simulink model "TosAeb"  (R2021a)
settings: Solver=FixedStepDiscrete  StartTime=0.0  StopTime=inf  FixedStep=0.01  RelTol=1e-3
undefined symbols (not defined in the file or in its folder): ACCModSts, AccSts1, AEB_ObjectMovingOrientation, AEB_ObjectMovingState, CN_BTCActive, CN_CurrentGearR, CN_CurrentGearR_DHT, CN_PTCActive, CN_VDCActive, CN_VSC_EspOff, CN_VSC_FCW_AEB_Error, CN_VSC_FCW_AEB_NoDispay, CN_VSC_FCW_AEB_PerfmDgdtn, CN_VSC_FCW_AEB_TempUnAvlbl, CN_VSC_VehStandstill, CNaccelAvoidMax, CNaccelAvoidMin, CNbrakePedalPress, CNcomfortStrLeftAccelMax, CNcomfortStrLeftAccelMin, CNcomfortStrRightAccelMax, CNcomfortStrRightAccelMin, CNDrvDoorOpen, CNDrvModeEco, CNDrvModeNorm, CNDrvModeOther, CNDrvModeSport, CNDrvSBRreminder, CNNull, CNobjInvalidID, CNPassDoorOpen, CNPassSBRreminder, CNPEBSAccelNegInf, CNPEBSAccelNull, CNPEBSTimeInf, CNPEBSTimeNull, CNSTOPMAX, CNSTOPNULL, CNsubjWidth, CNSwtOFF, CNSwtON, CNTTCMAX, CNTTCNULL, CNVehConfigAEBCycNone, CNVehConfigAEBNone, CNVehConfigAEBPedNone, CNVehConfigFCWNone, CNVehConfigJANone, CollisionSeverity, FALSE, FCWSnvty, JACycObjCalcInfoDefaultParams, JAPedObjCalcInfoDefaultParams, JAVehObjCalcInfoDefaultParams, latCycObjCalcInfoDefaultParams, latPedObjCalcInfoDefaultParams, longObjCalcInfoDefaultParams, ModSts, movingStatus, ObjectType ... (+1112)
libraries: simulink x3031, AEBSFunctionLib x50, Ta_Library x33, AEBSStateMachineLib x23, Mylibrary x12, simulink_extras x7, ObjSelect_lib x3, dspstat3 x2
(part 90 of 102)

== system TosAeb/AEB_Objsel/TA_Processor/AEB_ObjSelect/TargetInfoCheck/Data_precess/TA_EffectCalculate/Obj_FilterFlgCalculate/Long_ObjFilter/TTR_Calculate/CalculateTTRAndTTM_else/LongitudinalTTRComputer/CalculateTTI/StationaryObject/CheckIfSolutionExists1/SizeChecker ==
  Signal : Inport  OutDataTypeStr=DataTypeIn
      settings: AttributesFormatString=DT=%<OutDataTypeStr>
  Abs : Abs  SaturateOnIntegerOverflow=on
  LimitSource : Constant  Value=Limit  OutDataTypeStr=Inherit: Inherit via back propagation
      settings: AttributesFormatString=DT=%<OutDataTypeStr>
  Relational Operator : RelationalOperator  Operator=>  OutDataTypeStr=boolean  RndMeth=Nearest  InputSameDT=on
      settings: AttributesFormatString=InputSameDT=%<InputSameDT>
  TestOk : Outport  OutDataTypeStr=boolean
      settings: AttributesFormatString=DT=%<OutDataTypeStr>
  wires:
  Relational Operator/1 -> TestOk/1  "TestOk"
  Signal/1 -> Abs/1  "Signal"
  LimitSource/1 -> Relational Operator/2  "LimitSource"
  Abs/1 -> Relational Operator/1  "AbsoluteSignal"

== system TosAeb/AEB_Objsel/TA_Processor/AEB_ObjSelect/TargetInfoCheck/Data_precess/TA_EffectCalculate/Obj_FilterFlgCalculate/Long_ObjFilter/TTR_Calculate/CalculateTTRAndTTM_else/LongitudinalTTRComputer/CalculateTTI/StationaryObject/TimeToIntersectionComputer/Compare To Zero ==
  u : Inport
  Compare : RelationalOperator  Operator=<  OutDataTypeStr=boolean  RndMeth=Nearest  InputSameDT=on
  Constant : Constant  DisableCoverage=on  Value=0  OutDataTypeStr=Inherit: Inherit via back propagation  PreserveConstantTs=on
  y : Outport
  wires:
  Constant/1 -> Compare/2
  u/1 -> Compare/1
  Compare/1 -> y/1

== system TosAeb/AEB_Objsel/TA_Processor/AEB_ObjSelect/TargetInfoCheck/Data_precess/TA_EffectCalculate/Obj_FilterFlgCalculate/Long_ObjFilter/TTR_Calculate/CalculateTTRAndTTM_else/LongitudinalTTRComputer/CalculateTTI/StationaryObject/TimeToIntersectionComputer/SolutionChooser ==
  note: If ARel is negative, choose the largest solution.
      | Otherwise, choose the smallest solution.
  Solutions : Inport
  ARel : Inport  Port=2  OutDataTypeStr=single
      settings: AttributesFormatString=DT=%<OutDataTypeStr>
  Compare To Zero1 : Subsystem  mask(Compare To Zero){relop=<, OutDataTypeStr=boolean, ZeroCross=on}
      mask prompts: relop "Simulink:masks:Operator_MP"; OutDataTypeStr "Simulink:masks:OutputDataType_MP"; ZeroCross "Simulink:masks:EnableZerocrossingDetection_MP"
      mask help: Simulink:masks:CompareToZero_MD
      mask init: same as TosAeb/AEB_Objsel/TA_Processor/AEB_ObjSelect/TargetInfoCheck/Data_precess/TA_EffectCalculate/Obj_FilterFlgCalculate/Long_ObjFilter/TTR_Calculate/CalculateTTRAndTTM_else/ModifyTTRAtSameSpeedAndAcceleration/Compare To Zero
      mask TypeOptions relop: == | ~= | < | <= | >= | >
      mask TypeOptions OutDataTypeStr: Simulink:masks:uint8 | Simulink:masks:boolean
      mask Callback ZeroCross: sl('zeroCrossEnable', gcbh);
      mask Display: disp([relop,' 0'])
  Selector : Selector  InputPortWidth=2  IndexOptions=Index vector (dialog)  Indices=1  OutputSizes=1
  Selector1 : Selector  InputPortWidth=2  IndexOptions=Index vector (dialog)  Indices=2  OutputSizes=1
  Switch3 : Switch  Criteria=u2 ~= 0  InputSameDT=on
      settings: AttributesFormatString=InputSameDT=%<InputSameDT>
  Solution : Outport
  wires:
  Selector1/1 -> Switch3/3
  Solutions/1 -> [Solutions], Selector/1
  Selector/1 -> Switch3/1
  Switch3/1 -> Solution/1  "Solution"
  ARel/1 -> Compare To Zero1/1(u)
  Compare To Zero1/1(y) -> Switch3/2  "NegativeARel"
  [Solutions] -> Selector1/1

== system TosAeb/AEB_Objsel/TA_Processor/AEB_ObjSelect/TargetInfoCheck/Data_precess/TA_EffectCalculate/Obj_FilterFlgCalculate/Long_ObjFilter/TTR_Calculate/CalculateTTRAndTTM_else/LongitudinalTTRComputer/CalculateTTI/StationaryObject/TimeToIntersectionComputer1/Compare To Zero ==
  u : Inport
  Compare : RelationalOperator  Operator=<  OutDataTypeStr=boolean  RndMeth=Nearest  InputSameDT=on
  Constant : Constant  DisableCoverage=on  Value=0  OutDataTypeStr=Inherit: Inherit via back propagation  PreserveConstantTs=on
  y : Outport
  wires:
  Compare/1 -> y/1
  u/1 -> Compare/1
  Constant/1 -> Compare/2

== system TosAeb/AEB_Objsel/TA_Processor/AEB_ObjSelect/TargetInfoCheck/Data_precess/TA_EffectCalculate/Obj_FilterFlgCalculate/Long_ObjFilter/TTR_Calculate/CalculateTTRAndTTM_else/LongitudinalTTRComputer/CalculateTTI/StationaryObject/TimeToIntersectionComputer1/SolutionChooser ==
  note: If ARel is negative, choose the largest solution.
      | Otherwise, choose the smallest solution.
  Solutions : Inport
  ARel : Inport  Port=2  OutDataTypeStr=single
      settings: AttributesFormatString=DT=%<OutDataTypeStr>
  Compare To Zero1 : Subsystem  mask(Compare To Zero){relop=<, OutDataTypeStr=boolean, ZeroCross=on}
      mask prompts: relop "Simulink:masks:Operator_MP"; OutDataTypeStr "Simulink:masks:OutputDataType_MP"; ZeroCross "Simulink:masks:EnableZerocrossingDetection_MP"
      mask help: Simulink:masks:CompareToZero_MD
      mask init: same as TosAeb/AEB_Objsel/TA_Processor/AEB_ObjSelect/TargetInfoCheck/Data_precess/TA_EffectCalculate/Obj_FilterFlgCalculate/Long_ObjFilter/TTR_Calculate/CalculateTTRAndTTM_else/ModifyTTRAtSameSpeedAndAcceleration/Compare To Zero
      mask TypeOptions relop: == | ~= | < | <= | >= | >
      mask TypeOptions OutDataTypeStr: Simulink:masks:uint8 | Simulink:masks:boolean
      mask Callback ZeroCross: sl('zeroCrossEnable', gcbh);
      mask Display: disp([relop,' 0'])
  Selector : Selector  InputPortWidth=2  IndexOptions=Index vector (dialog)  Indices=1  OutputSizes=1
  Selector1 : Selector  InputPortWidth=2  IndexOptions=Index vector (dialog)  Indices=2  OutputSizes=1
  Switch3 : Switch  Criteria=u2 ~= 0  InputSameDT=on
      settings: AttributesFormatString=InputSameDT=%<InputSameDT>
  Solution : Outport
  wires:
  [Solutions] -> Selector1/1
  Compare To Zero1/1(y) -> Switch3/2  "NegativeARel"
  ARel/1 -> Compare To Zero1/1(u)
  Switch3/1 -> Solution/1  "Solution"
  Selector/1 -> Switch3/1
  Solutions/1 -> Selector/1, [Solutions]
  Selector1/1 -> Switch3/3

== system TosAeb/AEB_Objsel/TA_Processor/AEB_ObjSelect/TargetInfoCheck/Data_precess/TA_EffectCalculate/ValideateObj/ObjPos_Calculate/ObjPos_Calculateelse/InPath_Cal/DetermineInPath/PredictHostLateralPositionAtTTR/CalculateHeading/ExtractVelocitiesAtStartOfSegments/CheckSpeed ==
  x : Inport
  y : Inport  Port=2
  LatSpdThresholdNonVeh1 : Constant  Value=P_TA_SpdThdInAsyEvlrCritEve
  Product : Product
  Product1 : Product
  Product2 : Product
  Relational Operator : RelationalOperator  Operator=>  OutDataTypeStr=boolean  RndMeth=Nearest
  Subtract : Sum
  SpeedValid : Outport
  wires:
  y/1 -> Product/1, Product/2
  Subtract/1 -> Relational Operator/1  "VelocitySquared"
  Product1/1 -> Subtract/1
  Product/1 -> Subtract/2
  x/1 -> Product1/2, Product1/1
  LatSpdThresholdNonVeh1/1 -> Product2/1, Product2/2
  Product2/1 -> Relational Operator/2
  Relational Operator/1 -> SpeedValid/1  "SpeedValid"

== system TosAeb/AEB_Objsel/TA_Processor/AEB_ObjSelect/TargetInfoCheck/Data_precess/TA_EffectCalculate/ValideateObj/ObjPos_Calculate/ObjPos_Calculateelse/InPath_Cal/DetermineInPath/PredictHostLateralPositionAtTTR/CalculateHeading/ExtractVelocitiesAtStartOfSegments/ExtractVelocityVectorFirstPoint ==
  PathEstimn : Inport
  FirstPointInSegment : Constant  Value=single(0)
  PositionAndDerivative : MATLAB Function  TreatAsAtomicUnit=on
  PositionAndDerivative1 : MATLAB Function  TreatAsAtomicUnit=on
  SegmentComponents : Subsystem
  Terminator1 : Terminator
  Terminator2 : Terminator
  dx : Outport
  dy : Outport  Port=2
  wires:
  SegmentComponents/6(P1y) -> PositionAndDerivative1/2(P1)
  SegmentComponents/2(P1x) -> PositionAndDerivative/2(P1)
  SegmentComponents/7(P2y) -> PositionAndDerivative1/3(P2)
  SegmentComponents/1(P0x) -> PositionAndDerivative/1(P0)
  SegmentComponents/8(P3y) -> PositionAndDerivative1/4(P3)
  SegmentComponents/4(P3x) -> PositionAndDerivative/4(P3)
  SegmentComponents/3(P2x) -> PositionAndDerivative/3(P2)
  SegmentComponents/5(P0y) -> PositionAndDerivative1/1(P0)
  PathEstimn/1 -> SegmentComponents/1(Segment)
  PositionAndDerivative1/2(dy) -> dy/1
  PositionAndDerivative/1(x) -> Terminator1/1
  FirstPointInSegment/1 -> PositionAndDerivative1/5(tau), PositionAndDerivative/5(tau)
  PositionAndDerivative/2(dx) -> dx/1
  PositionAndDerivative1/1(y) -> Terminator2/1

== system TosAeb/FcwAebJa_Step_sys/ALG_AEBSystem/ALG_AEBController/AEB_LongTgtInfoCalc/LongObjCalcInfoCalc/AEBS_LongCollisionAssesApp/LongCollisionAvoidAssess/HighLvlBrkCalc/HighLvlBrkCalc4Brake/accelAvoidHighLvlBrkSituation2/accelAvoidHighLvlBrkCalc/If Action Subsystem1/accelAvoidLowLvlBrkCalc/Situation1/Case1 ==
  axAbsObj : Inport
  vx : Inport  Port=2
  dxEgoBrake : Inport  Port=3
  Action Port : ActionPort  ActionPortLabel=if(u1)
  Divide : Product  Inputs=*/
  Gain : Gain  Gain=2
  Math Function : Math  Operator=square  SignedPower=on  SaturateOnIntegerOverflow=on
  Subtract : Sum  Inputs=+-
  accelAvoid : Outport
  wires:
  vx/1 -> Math Function/1
  Math Function/1 -> Divide/1
  Gain/1 -> Divide/2
  dxEgoBrake/1 -> Gain/1
  Divide/1 -> Subtract/2
  axAbsObj/1 -> Subtract/1
  Subtract/1 -> accelAvoid/1

== system TosAeb/FcwAebJa_Step_sys/ALG_AEBSystem/ALG_AEBController/AEB_LongTgtInfoCalc/LongObjCalcInfoCalc/AEBS_LongCollisionAssesApp/LongCollisionAvoidAssess/HighLvlBrkCalc/HighLvlBrkCalc4Brake/accelAvoidHighLvlBrkSituation2/accelAvoidHighLvlBrkCalc/If Action Subsystem1/accelAvoidLowLvlBrkCalc/Situation1/Case2 ==
  note: vEgo^2
      | aAvoid = ----------------------------------
      |                   vObj^2
      |                  ---------  - 2(dRel - dScure)
      |                   aObj
  subjVel : Inport
  vxAbsObj : Inport  Port=2
  axAbsObj : Inport  Port=3
  dxEgoBrake : Inport  Port=4
  Action Port : ActionPort  ActionPortLabel=else
  Divide : Product  Inputs=*/
  Divide1 : Product  Inputs=*/
  Gain : Gain  Gain=2
  Math Function : Math  Operator=square  SignedPower=on  SaturateOnIntegerOverflow=on
  Math Function1 : Math  Operator=square  SignedPower=on  SaturateOnIntegerOverflow=on
  Subtract : Sum  Inputs=+-
  accelAvoid : Outport
  wires:
  vxAbsObj/1 -> Math Function/1
  Math Function/1 -> Divide/1
  axAbsObj/1 -> Divide/2
  Divide/1 -> Subtract/1
  dxEgoBrake/1 -> Gain/1
  Gain/1 -> Subtract/2
  subjVel/1 -> Math Function1/1
  Subtract/1 -> Divide1/2
  Math Function1/1 -> Divide1/1
  Divide1/1 -> accelAvoid/1

== system TosAeb/FcwAebJa_Step_sys/ALG_AEBSystem/ALG_AEBController/AEB_LongTgtInfoCalc/LongObjCalcInfoCalc/AEBS_LongCollisionAssesApp/LongCollisionAvoidAssess/HighLvlBrkCalc/HighLvlBrkCalc4Brake/accelAvoidHighLvlBrkSituation2/accelAvoidHighLvlBrkCalc/If Action Subsystem1/accelAvoidLowLvlBrkCalc/Situation1/collisionFirstFlgCalc ==
  note: Calculate Ego and Obj Collision Time and
      |  Determine if the Collision Time is less than the object stop time
  dxEgoBrake : Inport
  vx : Inport  Port=2
  tObjStop : Inport  Port=3
  CNTTCMAX : Constant  Value=CNTTCMAX
  Divide : Product  Inputs=*/
  Gain : Gain  Gain=-1
  Gain1 : Gain  Gain=2
  MinMax : MinMax  Inputs=2
  Relational Operator : RelationalOperator  Operator=<  OutDataTypeStr=boolean  RndMeth=Simplest
  collisionFirstFlg : Outport
  wires:
  Divide/1 -> Gain1/1
  dxEgoBrake/1 -> Divide/1
  vx/1 -> Gain/1
  Gain/1 -> Divide/2
  Gain1/1 -> MinMax/1
  tObjStop/1 -> Relational Operator/2
  Relational Operator/1 -> collisionFirstFlg/1  "collisionFirstFlg"
  MinMax/1 -> Relational Operator/1
  CNTTCMAX/1 -> MinMax/2

== system TosAeb/FcwAebJa_Step_sys/ALG_AEBSystem/ALG_AEBController/AEB_LongTgtInfoCalc/LongObjCalcInfoCalc/AEBS_LongCollisionAssesApp/LongCollisionAvoidAssess/HighLvlBrkCalc/HighLvlBrkCalc4Brake/accelAvoidHighLvlBrkSituation2/accelAvoidHighLvlBrkCalc/If Action Subsystem1/accelAvoidLowLvlBrkCalc1/Situation1/Case1 ==
  axAbsObj : Inport
  vx : Inport  Port=2
  dxEgoBrake : Inport  Port=3
  Action Port : ActionPort
  Divide : Product  Inputs=*/
  Gain : Gain  Gain=2
  Math Function : Math  Operator=square  SignedPower=on  SaturateOnIntegerOverflow=on
  Subtract : Sum  Inputs=+-
  accelAvoid : Outport
  wires:
  Subtract/1 -> accelAvoid/1
  axAbsObj/1 -> Subtract/1
  Divide/1 -> Subtract/2
  dxEgoBrake/1 -> Gain/1
  Gain/1 -> Divide/2
  Math Function/1 -> Divide/1
  vx/1 -> Math Function/1

== system TosAeb/FcwAebJa_Step_sys/ALG_AEBSystem/ALG_AEBController/AEB_LongTgtInfoCalc/LongObjCalcInfoCalc/AEBS_LongCollisionAssesApp/LongCollisionAvoidAssess/HighLvlBrkCalc/HighLvlBrkCalc4Brake/accelAvoidHighLvlBrkSituation2/accelAvoidHighLvlBrkCalc/If Action Subsystem1/accelAvoidLowLvlBrkCalc1/Situation1/Case2 ==
  note: vEgo^2
      | aAvoid = ----------------------------------
      |                   vObj^2
      |                  ---------  - 2(dRel - dScure)
      |                   aObj
  subjVel : Inport
  vxAbsObj : Inport  Port=2
  axAbsObj : Inport  Port=3
  dxEgoBrake : Inport  Port=4
  Action Port : ActionPort
  Divide : Product  Inputs=*/
  Divide1 : Product  Inputs=*/
  Gain : Gain  Gain=2
  Math Function : Math  Operator=square  SignedPower=on  SaturateOnIntegerOverflow=on
  Math Function1 : Math  Operator=square  SignedPower=on  SaturateOnIntegerOverflow=on
  Subtract : Sum  Inputs=+-
  accelAvoid : Outport
  wires:
  Divide1/1 -> accelAvoid/1
  Math Function1/1 -> Divide1/1
  Subtract/1 -> Divide1/2
  subjVel/1 -> Math Function1/1
  Gain/1 -> Subtract/2
  dxEgoBrake/1 -> Gain/1
  Divide/1 -> Subtract/1
  axAbsObj/1 -> Divide/2
  Math Function/1 -> Divide/1
  vxAbsObj/1 -> Math Function/1

== system TosAeb/FcwAebJa_Step_sys/ALG_AEBSystem/ALG_AEBController/AEB_LongTgtInfoCalc/LongObjCalcInfoCalc/AEBS_LongCollisionAssesApp/LongCollisionAvoidAssess/HighLvlBrkCalc/HighLvlBrkCalc4Brake/accelAvoidHighLvlBrkSituation2/accelAvoidHighLvlBrkCalc/If Action Subsystem1/accelAvoidLowLvlBrkCalc1/Situation1/collisionFirstFlgCalc ==
  note: Calculate Ego and Obj Collision Time and
      |  Determine if the Collision Time is less than the object stop time
  dxEgoBrake : Inport
  vx : Inport  Port=2
  tObjStop : Inport  Port=3
  Divide : Product  Inputs=*/
  Gain : Gain  Gain=-1
  Gain1 : Gain  Gain=2
  Relational Operator : RelationalOperator  Operator=<  OutDataTypeStr=boolean  RndMeth=Simplest
  Saturation : Saturate  UpperLimit=CNTTCMAX  LowerLimit=CNTTCNULL
      settings: AttributesFormatString=|
      | UpperLimit:%<UpperLimit>
      | LowerLimit:%<LowerLimit>
  collisionFirstFlg : Outport
  wires:
  Saturation/1 -> Relational Operator/1
  Relational Operator/1 -> collisionFirstFlg/1
  tObjStop/1 -> Relational Operator/2
  Gain1/1 -> Saturation/1
  Gain/1 -> Divide/2
  vx/1 -> Gain/1
  dxEgoBrake/1 -> Divide/1
  Divide/1 -> Gain1/1

== system TosAeb/FcwAebJa_Step_sys/ALG_AEBSystem/ALG_AEBController/AEB_LongTgtInfoCalc/LongObjCalcInfoCalc/AEBS_LongCollisionAssesApp/LongCollisionAvoidAssess/HighLvlBrkCalc/HighLvlBrkCalc4Brake/accelAvoidHighLvlBrkSituation3/If Action Subsystem1/accelAvoidHighLvlBrkCalc/accelAvoidLowLvlBrkCalc/Situation1/Case1 ==
  axAbsObj : Inport
  vx : Inport  Port=2
  dxEgoBrake : Inport  Port=3
  Action Port : ActionPort  ActionPortLabel=if(u1)
  Divide : Product  Inputs=*/
  Gain : Gain  Gain=2
  Math Function : Math  Operator=square  SignedPower=on  SaturateOnIntegerOverflow=on
  Subtract : Sum  Inputs=+-
  accelAvoid : Outport
  wires:
  vx/1 -> Math Function/1
  Math Function/1 -> Divide/1
  Gain/1 -> Divide/2
  dxEgoBrake/1 -> Gain/1
  Divide/1 -> Subtract/2
  axAbsObj/1 -> Subtract/1
  Subtract/1 -> accelAvoid/1

== system TosAeb/FcwAebJa_Step_sys/ALG_AEBSystem/ALG_AEBController/AEB_LongTgtInfoCalc/LongObjCalcInfoCalc/AEBS_LongCollisionAssesApp/LongCollisionAvoidAssess/HighLvlBrkCalc/HighLvlBrkCalc4Brake/accelAvoidHighLvlBrkSituation3/If Action Subsystem1/accelAvoidHighLvlBrkCalc/accelAvoidLowLvlBrkCalc/Situation1/Case2 ==
  note: vEgo^2
      | aAvoid = ----------------------------------
      |                   vObj^2
      |                  ---------  - 2(dRel - dScure)
      |                   aObj
  subjVel : Inport
  vxAbsObj : Inport  Port=2
  axAbsObj : Inport  Port=3
  dxEgoBrake : Inport  Port=4
  Action Port : ActionPort  ActionPortLabel=else
  Divide : Product  Inputs=*/
  Divide1 : Product  Inputs=*/
  Gain : Gain  Gain=2
  Math Function : Math  Operator=square  SignedPower=on  SaturateOnIntegerOverflow=on
  Math Function1 : Math  Operator=square  SignedPower=on  SaturateOnIntegerOverflow=on
  Subtract : Sum  Inputs=+-
  accelAvoid : Outport
  wires:
  vxAbsObj/1 -> Math Function/1
  Math Function/1 -> Divide/1
  axAbsObj/1 -> Divide/2
  Divide/1 -> Subtract/1
  dxEgoBrake/1 -> Gain/1
  Gain/1 -> Subtract/2
  subjVel/1 -> Math Function1/1
  Subtract/1 -> Divide1/2
  Math Function1/1 -> Divide1/1
  Divide1/1 -> accelAvoid/1

== system TosAeb/FcwAebJa_Step_sys/ALG_AEBSystem/ALG_AEBController/AEB_LongTgtInfoCalc/LongObjCalcInfoCalc/AEBS_LongCollisionAssesApp/LongCollisionAvoidAssess/HighLvlBrkCalc/HighLvlBrkCalc4Brake/accelAvoidHighLvlBrkSituation3/If Action Subsystem1/accelAvoidHighLvlBrkCalc/accelAvoidLowLvlBrkCalc/Situation1/collisionFirstFlgCalc ==
  note: Calculate Ego and Obj Collision Time and
      |  Determine if the Collision Time is less than the object stop time
  dxEgoBrake : Inport
  vx : Inport  Port=2
  tObjStop : Inport  Port=3
  CNTTCMAX : Constant  Value=CNTTCMAX
  Divide : Product  Inputs=*/
  Gain : Gain  Gain=-1
  Gain1 : Gain  Gain=2
  MinMax : MinMax  Inputs=2
  Relational Operator : RelationalOperator  Operator=<  OutDataTypeStr=boolean  RndMeth=Simplest
  collisionFirstFlg : Outport
  wires:
  Divide/1 -> Gain1/1
  dxEgoBrake/1 -> Divide/1
  vx/1 -> Gain/1
  Gain/1 -> Divide/2
  Gain1/1 -> MinMax/1
  tObjStop/1 -> Relational Operator/2
  Relational Operator/1 -> collisionFirstFlg/1  "collisionFirstFlg"
  MinMax/1 -> Relational Operator/1
  CNTTCMAX/1 -> MinMax/2

== system TosAeb/FcwAebJa_Step_sys/ALG_AEBSystem/ALG_AEBController/AEB_LongTgtInfoCalc/LongObjCalcInfoCalc/AEBS_LongCollisionAssesApp/LongCollisionAvoidAssess/HighLvlBrkCalc/HighLvlBrkCalc4Brake/accelAvoidHighLvlBrkSituation3/If Action Subsystem1/accelAvoidHighLvlBrkCalc/accelAvoidLowLvlBrkCalc1/Situation1/Case1 ==
  axAbsObj : Inport
  vx : Inport  Port=2
  dxEgoBrake : Inport  Port=3
  Action Port : ActionPort
  Divide : Product  Inputs=*/
  Gain : Gain  Gain=2
  Math Function : Math  Operator=square  SignedPower=on  SaturateOnIntegerOverflow=on
  Subtract : Sum  Inputs=+-
  accelAvoid : Outport
  wires:
  Subtract/1 -> accelAvoid/1
  axAbsObj/1 -> Subtract/1
  Divide/1 -> Subtract/2
  dxEgoBrake/1 -> Gain/1
  Gain/1 -> Divide/2
  Math Function/1 -> Divide/1
  vx/1 -> Math Function/1

== system TosAeb/FcwAebJa_Step_sys/ALG_AEBSystem/ALG_AEBController/AEB_LongTgtInfoCalc/LongObjCalcInfoCalc/AEBS_LongCollisionAssesApp/LongCollisionAvoidAssess/HighLvlBrkCalc/HighLvlBrkCalc4Brake/accelAvoidHighLvlBrkSituation3/If Action Subsystem1/accelAvoidHighLvlBrkCalc/accelAvoidLowLvlBrkCalc1/Situation1/Case2 ==
  note: vEgo^2
      | aAvoid = ----------------------------------
      |                   vObj^2
      |                  ---------  - 2(dRel - dScure)
      |                   aObj
  subjVel : Inport
  vxAbsObj : Inport  Port=2
  axAbsObj : Inport  Port=3
  dxEgoBrake : Inport  Port=4
  Action Port : ActionPort
  Divide : Product  Inputs=*/
  Divide1 : Product  Inputs=*/
  Gain : Gain  Gain=2
  Math Function : Math  Operator=square  SignedPower=on  SaturateOnIntegerOverflow=on
  Math Function1 : Math  Operator=square  SignedPower=on  SaturateOnIntegerOverflow=on
  Subtract : Sum  Inputs=+-
  accelAvoid : Outport
  wires:
  Divide1/1 -> accelAvoid/1
  Math Function1/1 -> Divide1/1
  Subtract/1 -> Divide1/2
  subjVel/1 -> Math Function1/1
  Gain/1 -> Subtract/2
  dxEgoBrake/1 -> Gain/1
  Divide/1 -> Subtract/1
  axAbsObj/1 -> Divide/2
  Math Function/1 -> Divide/1
  vxAbsObj/1 -> Math Function/1

== system TosAeb/FcwAebJa_Step_sys/ALG_AEBSystem/ALG_AEBController/AEB_LongTgtInfoCalc/LongObjCalcInfoCalc/AEBS_LongCollisionAssesApp/LongCollisionAvoidAssess/HighLvlBrkCalc/HighLvlBrkCalc4Brake/accelAvoidHighLvlBrkSituation3/If Action Subsystem1/accelAvoidHighLvlBrkCalc/accelAvoidLowLvlBrkCalc1/Situation1/collisionFirstFlgCalc ==
  note: Calculate Ego and Obj Collision Time and
      |  Determine if the Collision Time is less than the object stop time
  dxEgoBrake : Inport
  vx : Inport  Port=2
  tObjStop : Inport  Port=3
  Divide : Product  Inputs=*/
  Gain : Gain  Gain=-1
  Gain1 : Gain  Gain=2
  Relational Operator : RelationalOperator  Operator=<  OutDataTypeStr=boolean  RndMeth=Simplest
  Saturation : Saturate  UpperLimit=CNTTCMAX  LowerLimit=CNTTCNULL
      settings: AttributesFormatString=|
      | UpperLimit:%<UpperLimit>
      | LowerLimit:%<LowerLimit>
  collisionFirstFlg : Outport
  wires:
  Saturation/1 -> Relational Operator/1
  Relational Operator/1 -> collisionFirstFlg/1
  tObjStop/1 -> Relational Operator/2
  Gain1/1 -> Saturation/1
  Gain/1 -> Divide/2
  vx/1 -> Gain/1
  dxEgoBrake/1 -> Divide/1
  Divide/1 -> Gain1/1

== system TosAeb/FcwAebJa_Step_sys/ALG_AEBSystem/ALG_AEBController/AEB_LongTgtInfoCalc/LongObjCalcInfoCalc/AEBS_LongCollisionAssesApp/LongCollisionAvoidAssess/HighLvlBrkCalc/HighLvlBrkCalc4Warning/accelAvoidHighLvlBrkSituation2/accelAvoidHighLvlBrkCalc/If Action Subsystem1/accelAvoidLowLvlBrkCalc/Situation1/Case1 ==
  axAbsObj : Inport
  vx : Inport  Port=2
  dxEgoBrake : Inport  Port=3
  Action Port : ActionPort  ActionPortLabel=if(u1)
  Divide : Product  Inputs=*/
  Gain : Gain  Gain=2
  Math Function : Math  Operator=square  SignedPower=on  SaturateOnIntegerOverflow=on
  Subtract : Sum  Inputs=+-
  accelAvoid : Outport
  wires:
  Subtract/1 -> accelAvoid/1
  axAbsObj/1 -> Subtract/1
  Divide/1 -> Subtract/2
  dxEgoBrake/1 -> Gain/1
  Gain/1 -> Divide/2
  Math Function/1 -> Divide/1
  vx/1 -> Math Function/1

== system TosAeb/FcwAebJa_Step_sys/ALG_AEBSystem/ALG_AEBController/AEB_LongTgtInfoCalc/LongObjCalcInfoCalc/AEBS_LongCollisionAssesApp/LongCollisionAvoidAssess/HighLvlBrkCalc/HighLvlBrkCalc4Warning/accelAvoidHighLvlBrkSituation2/accelAvoidHighLvlBrkCalc/If Action Subsystem1/accelAvoidLowLvlBrkCalc/Situation1/Case2 ==
  note: vEgo^2
      | aAvoid = ----------------------------------
      |                   vObj^2
      |                  ---------  - 2(dRel - dScure)
      |                   aObj
  subjVel : Inport
  vxAbsObj : Inport  Port=2
  axAbsObj : Inport  Port=3
  dxEgoBrake : Inport  Port=4
  Action Port : ActionPort  ActionPortLabel=else
  Divide : Product  Inputs=*/
  Divide1 : Product  Inputs=*/
  Gain : Gain  Gain=2
  Math Function : Math  Operator=square  SignedPower=on  SaturateOnIntegerOverflow=on
  Math Function1 : Math  Operator=square  SignedPower=on  SaturateOnIntegerOverflow=on
  Subtract : Sum  Inputs=+-
  accelAvoid : Outport
  wires:
  Divide1/1 -> accelAvoid/1
  Math Function1/1 -> Divide1/1
  Subtract/1 -> Divide1/2
  subjVel/1 -> Math Function1/1
  Gain/1 -> Subtract/2
  dxEgoBrake/1 -> Gain/1
  Divide/1 -> Subtract/1
  axAbsObj/1 -> Divide/2
  Math Function/1 -> Divide/1
  vxAbsObj/1 -> Math Function/1

== system TosAeb/FcwAebJa_Step_sys/ALG_AEBSystem/ALG_AEBController/AEB_LongTgtInfoCalc/LongObjCalcInfoCalc/AEBS_LongCollisionAssesApp/LongCollisionAvoidAssess/HighLvlBrkCalc/HighLvlBrkCalc4Warning/accelAvoidHighLvlBrkSituation2/accelAvoidHighLvlBrkCalc/If Action Subsystem1/accelAvoidLowLvlBrkCalc/Situation1/collisionFirstFlgCalc ==
  note: Calculate Ego and Obj Collision Time and
      |  Determine if the Collision Time is less than the object stop time
  dxEgoBrake : Inport
  vx : Inport  Port=2
  tObjStop : Inport  Port=3
  CNTTCMAX : Constant  Value=CNTTCMAX
  Divide : Product  Inputs=*/
  Gain : Gain  Gain=-1
  Gain1 : Gain  Gain=2
  MinMax : MinMax  Inputs=2
  Relational Operator : RelationalOperator  Operator=<  OutDataTypeStr=boolean  RndMeth=Simplest
  collisionFirstFlg : Outport
  wires:
  CNTTCMAX/1 -> MinMax/2
  MinMax/1 -> Relational Operator/1
  Relational Operator/1 -> collisionFirstFlg/1  "collisionFirstFlg"
  tObjStop/1 -> Relational Operator/2
  Gain1/1 -> MinMax/1
  Gain/1 -> Divide/2
  vx/1 -> Gain/1
  dxEgoBrake/1 -> Divide/1
  Divide/1 -> Gain1/1

== system TosAeb/FcwAebJa_Step_sys/ALG_AEBSystem/ALG_AEBController/AEB_LongTgtInfoCalc/LongObjCalcInfoCalc/AEBS_LongCollisionAssesApp/LongCollisionAvoidAssess/HighLvlBrkCalc/HighLvlBrkCalc4Warning/accelAvoidHighLvlBrkSituation2/accelAvoidHighLvlBrkCalc/If Action Subsystem1/accelAvoidLowLvlBrkCalc1/Situation1/Case1 ==
  axAbsObj : Inport
  vx : Inport  Port=2
  dxEgoBrake : Inport  Port=3
  Action Port : ActionPort
  Divide : Product  Inputs=*/
  Gain : Gain  Gain=2
  Math Function : Math  Operator=square  SignedPower=on  SaturateOnIntegerOverflow=on
  Subtract : Sum  Inputs=+-
  accelAvoid : Outport
  wires:
  vx/1 -> Math Function/1
  Math Function/1 -> Divide/1
  Gain/1 -> Divide/2
  dxEgoBrake/1 -> Gain/1
  Divide/1 -> Subtract/2
  axAbsObj/1 -> Subtract/1
  Subtract/1 -> accelAvoid/1

== system TosAeb/FcwAebJa_Step_sys/ALG_AEBSystem/ALG_AEBController/AEB_LongTgtInfoCalc/LongObjCalcInfoCalc/AEBS_LongCollisionAssesApp/LongCollisionAvoidAssess/HighLvlBrkCalc/HighLvlBrkCalc4Warning/accelAvoidHighLvlBrkSituation2/accelAvoidHighLvlBrkCalc/If Action Subsystem1/accelAvoidLowLvlBrkCalc1/Situation1/Case2 ==
  note: vEgo^2
      | aAvoid = ----------------------------------
      |                   vObj^2
      |                  ---------  - 2(dRel - dScure)
      |                   aObj
  subjVel : Inport
  vxAbsObj : Inport  Port=2
  axAbsObj : Inport  Port=3
  dxEgoBrake : Inport  Port=4
  Action Port : ActionPort
  Divide : Product  Inputs=*/
  Divide1 : Product  Inputs=*/
  Gain : Gain  Gain=2
  Math Function : Math  Operator=square  SignedPower=on  SaturateOnIntegerOverflow=on
  Math Function1 : Math  Operator=square  SignedPower=on  SaturateOnIntegerOverflow=on
  Subtract : Sum  Inputs=+-
  accelAvoid : Outport
  wires:
  vxAbsObj/1 -> Math Function/1
  Math Function/1 -> Divide/1
  axAbsObj/1 -> Divide/2
  Divide/1 -> Subtract/1
  dxEgoBrake/1 -> Gain/1
  Gain/1 -> Subtract/2
  subjVel/1 -> Math Function1/1
  Subtract/1 -> Divide1/2
  Math Function1/1 -> Divide1/1
  Divide1/1 -> accelAvoid/1

== system TosAeb/FcwAebJa_Step_sys/ALG_AEBSystem/ALG_AEBController/AEB_LongTgtInfoCalc/LongObjCalcInfoCalc/AEBS_LongCollisionAssesApp/LongCollisionAvoidAssess/HighLvlBrkCalc/HighLvlBrkCalc4Warning/accelAvoidHighLvlBrkSituation2/accelAvoidHighLvlBrkCalc/If Action Subsystem1/accelAvoidLowLvlBrkCalc1/Situation1/collisionFirstFlgCalc ==
  note: Calculate Ego and Obj Collision Time and
      |  Determine if the Collision Time is less than the object stop time
  dxEgoBrake : Inport
  vx : Inport  Port=2
  tObjStop : Inport  Port=3
  Divide : Product  Inputs=*/
  Gain : Gain  Gain=-1
  Gain1 : Gain  Gain=2
  Relational Operator : RelationalOperator  Operator=<  OutDataTypeStr=boolean  RndMeth=Simplest
  Saturation : Saturate  UpperLimit=CNTTCMAX  LowerLimit=CNTTCNULL
      settings: AttributesFormatString=|
      | UpperLimit:%<UpperLimit>
      | LowerLimit:%<LowerLimit>
  collisionFirstFlg : Outport
  wires:
  Divide/1 -> Gain1/1
  dxEgoBrake/1 -> Divide/1
  vx/1 -> Gain/1
  Gain/1 -> Divide/2
  Gain1/1 -> Saturation/1
  tObjStop/1 -> Relational Operator/2
  Relational Operator/1 -> collisionFirstFlg/1
  Saturation/1 -> Relational Operator/1

== system TosAeb/FcwAebJa_Step_sys/ALG_AEBSystem/ALG_AEBController/AEB_LongTgtInfoCalc/LongObjCalcInfoCalc/AEBS_LongCollisionAssesApp/LongCollisionAvoidAssess/HighLvlBrkCalc/HighLvlBrkCalc4Warning/accelAvoidHighLvlBrkSituation3/If Action Subsystem1/accelAvoidHighLvlBrkCalc/accelAvoidLowLvlBrkCalc/Situation1/Case1 ==
  axAbsObj : Inport
  vx : Inport  Port=2
  dxEgoBrake : Inport  Port=3
  Action Port : ActionPort  ActionPortLabel=if(u1)
  Divide : Product  Inputs=*/
  Gain : Gain  Gain=2
  Math Function : Math  Operator=square  SignedPower=on  SaturateOnIntegerOverflow=on
  Subtract : Sum  Inputs=+-
  accelAvoid : Outport
  wires:
  Subtract/1 -> accelAvoid/1
  axAbsObj/1 -> Subtract/1
  Divide/1 -> Subtract/2
  dxEgoBrake/1 -> Gain/1
  Gain/1 -> Divide/2
  Math Function/1 -> Divide/1
  vx/1 -> Math Function/1

== system TosAeb/FcwAebJa_Step_sys/ALG_AEBSystem/ALG_AEBController/AEB_LongTgtInfoCalc/LongObjCalcInfoCalc/AEBS_LongCollisionAssesApp/LongCollisionAvoidAssess/HighLvlBrkCalc/HighLvlBrkCalc4Warning/accelAvoidHighLvlBrkSituation3/If Action Subsystem1/accelAvoidHighLvlBrkCalc/accelAvoidLowLvlBrkCalc/Situation1/Case2 ==
  note: vEgo^2
      | aAvoid = ----------------------------------
      |                   vObj^2
      |                  ---------  - 2(dRel - dScure)
      |                   aObj
  subjVel : Inport
  vxAbsObj : Inport  Port=2
  axAbsObj : Inport  Port=3
  dxEgoBrake : Inport  Port=4
  Action Port : ActionPort  ActionPortLabel=else
  Divide : Product  Inputs=*/
  Divide1 : Product  Inputs=*/
  Gain : Gain  Gain=2
  Math Function : Math  Operator=square  SignedPower=on  SaturateOnIntegerOverflow=on
  Math Function1 : Math  Operator=square  SignedPower=on  SaturateOnIntegerOverflow=on
  Subtract : Sum  Inputs=+-
  accelAvoid : Outport
  wires:
  Divide1/1 -> accelAvoid/1
  Math Function1/1 -> Divide1/1
  Subtract/1 -> Divide1/2
  subjVel/1 -> Math Function1/1
  Gain/1 -> Subtract/2
  dxEgoBrake/1 -> Gain/1
  Divide/1 -> Subtract/1
  axAbsObj/1 -> Divide/2
  Math Function/1 -> Divide/1
  vxAbsObj/1 -> Math Function/1

== system TosAeb/FcwAebJa_Step_sys/ALG_AEBSystem/ALG_AEBController/AEB_LongTgtInfoCalc/LongObjCalcInfoCalc/AEBS_LongCollisionAssesApp/LongCollisionAvoidAssess/HighLvlBrkCalc/HighLvlBrkCalc4Warning/accelAvoidHighLvlBrkSituation3/If Action Subsystem1/accelAvoidHighLvlBrkCalc/accelAvoidLowLvlBrkCalc/Situation1/collisionFirstFlgCalc ==
  note: Calculate Ego and Obj Collision Time and
      |  Determine if the Collision Time is less than the object stop time
  dxEgoBrake : Inport
  vx : Inport  Port=2
  tObjStop : Inport  Port=3
  CNTTCMAX : Constant  Value=CNTTCMAX
  Divide : Product  Inputs=*/
  Gain : Gain  Gain=-1
  Gain1 : Gain  Gain=2
  MinMax : MinMax  Inputs=2
  Relational Operator : RelationalOperator  Operator=<  OutDataTypeStr=boolean  RndMeth=Simplest
  collisionFirstFlg : Outport
  wires:
  CNTTCMAX/1 -> MinMax/2
  MinMax/1 -> Relational Operator/1
  Relational Operator/1 -> collisionFirstFlg/1  "collisionFirstFlg"
  tObjStop/1 -> Relational Operator/2
  Gain1/1 -> MinMax/1
  Gain/1 -> Divide/2
  vx/1 -> Gain/1
  dxEgoBrake/1 -> Divide/1
  Divide/1 -> Gain1/1

== system TosAeb/FcwAebJa_Step_sys/ALG_AEBSystem/ALG_AEBController/AEB_LongTgtInfoCalc/LongObjCalcInfoCalc/AEBS_LongCollisionAssesApp/LongCollisionAvoidAssess/HighLvlBrkCalc/HighLvlBrkCalc4Warning/accelAvoidHighLvlBrkSituation3/If Action Subsystem1/accelAvoidHighLvlBrkCalc/accelAvoidLowLvlBrkCalc1/Situation1/Case1 ==
  axAbsObj : Inport
  vx : Inport  Port=2
  dxEgoBrake : Inport  Port=3
  Action Port : ActionPort
  Divide : Product  Inputs=*/
  Gain : Gain  Gain=2
  Math Function : Math  Operator=square  SignedPower=on  SaturateOnIntegerOverflow=on
  Subtract : Sum  Inputs=+-
  accelAvoid : Outport
  wires:
  vx/1 -> Math Function/1
  Math Function/1 -> Divide/1
  Gain/1 -> Divide/2
  dxEgoBrake/1 -> Gain/1
  Divide/1 -> Subtract/2
  axAbsObj/1 -> Subtract/1
  Subtract/1 -> accelAvoid/1

== system TosAeb/FcwAebJa_Step_sys/ALG_AEBSystem/ALG_AEBController/AEB_LongTgtInfoCalc/LongObjCalcInfoCalc/AEBS_LongCollisionAssesApp/LongCollisionAvoidAssess/HighLvlBrkCalc/HighLvlBrkCalc4Warning/accelAvoidHighLvlBrkSituation3/If Action Subsystem1/accelAvoidHighLvlBrkCalc/accelAvoidLowLvlBrkCalc1/Situation1/Case2 ==
  note: vEgo^2
      | aAvoid = ----------------------------------
      |                   vObj^2
      |                  ---------  - 2(dRel - dScure)
      |                   aObj
  subjVel : Inport
  vxAbsObj : Inport  Port=2
  axAbsObj : Inport  Port=3
  dxEgoBrake : Inport  Port=4
  Action Port : ActionPort
  Divide : Product  Inputs=*/
  Divide1 : Product  Inputs=*/
  Gain : Gain  Gain=2
  Math Function : Math  Operator=square  SignedPower=on  SaturateOnIntegerOverflow=on
  Math Function1 : Math  Operator=square  SignedPower=on  SaturateOnIntegerOverflow=on
  Subtract : Sum  Inputs=+-
  accelAvoid : Outport
  wires:
  vxAbsObj/1 -> Math Function/1
  Math Function/1 -> Divide/1
  axAbsObj/1 -> Divide/2
  Divide/1 -> Subtract/1
  dxEgoBrake/1 -> Gain/1
  Gain/1 -> Subtract/2
  subjVel/1 -> Math Function1/1
  Subtract/1 -> Divide1/2
  Math Function1/1 -> Divide1/1
  Divide1/1 -> accelAvoid/1

== system TosAeb/FcwAebJa_Step_sys/ALG_AEBSystem/ALG_AEBController/AEB_LongTgtInfoCalc/LongObjCalcInfoCalc/AEBS_LongCollisionAssesApp/LongCollisionAvoidAssess/HighLvlBrkCalc/HighLvlBrkCalc4Warning/accelAvoidHighLvlBrkSituation3/If Action Subsystem1/accelAvoidHighLvlBrkCalc/accelAvoidLowLvlBrkCalc1/Situation1/collisionFirstFlgCalc ==
  note: Calculate Ego and Obj Collision Time and
      |  Determine if the Collision Time is less than the object stop time
  dxEgoBrake : Inport
  vx : Inport  Port=2
  tObjStop : Inport  Port=3
  Divide : Product  Inputs=*/
  Gain : Gain  Gain=-1
  Gain1 : Gain  Gain=2
  Relational Operator : RelationalOperator  Operator=<  OutDataTypeStr=boolean  RndMeth=Simplest
  Saturation : Saturate  UpperLimit=CNTTCMAX  LowerLimit=CNTTCNULL
      settings: AttributesFormatString=|
      | UpperLimit:%<UpperLimit>
      | LowerLimit:%<LowerLimit>
  collisionFirstFlg : Outport
  wires:
  Divide/1 -> Gain1/1
  dxEgoBrake/1 -> Divide/1
  vx/1 -> Gain/1
  Gain/1 -> Divide/2
  Gain1/1 -> Saturation/1
  tObjStop/1 -> Relational Operator/2
  Relational Operator/1 -> collisionFirstFlg/1
  Saturation/1 -> Relational Operator/1

== system TosAeb/AEB_Objsel/TA_Processor/AEB_ObjSelect/TargetInfoCheck/Data_precess/TA_EffectCalculate/Obj_FilterFlgCalculate/Long_ObjFilter/OverlapCalculate/BoudingBoxCalculate_Else/PosnatTTI/PredictHostPositionAtTTR/CalculateHeading/ExtractVelocitiesAtStartOfSegments/ExtractVelocityVectorFirstPoint/SegmentComponents ==
  Segment : Inport
  Bus Selector : BusSelector  OutputSignals=CtrlAryLgt,CtrlAryLat
  Selector : Selector  InputPortWidth=4  IndexOptions=Index vector (dialog)  Indices=1  OutputSizes=1
  Selector1 : Selector  InputPortWidth=4  IndexOptions=Index vector (dialog)  Indices=2  OutputSizes=1
  Selector2 : Selector  InputPortWidth=4  IndexOptions=Index vector (dialog)  Indices=3  OutputSizes=1
  Selector3 : Selector  InputPortWidth=4  IndexOptions=Index vector (dialog)  Indices=4  OutputSizes=1
  Selector4 : Selector  InputPortWidth=4  IndexOptions=Index vector (dialog)  Indices=1  OutputSizes=1
  Selector5 : Selector  InputPortWidth=4  IndexOptions=Index vector (dialog)  Indices=2  OutputSizes=1
  Selector6 : Selector  InputPortWidth=4  IndexOptions=Index vector (dialog)  Indices=3  OutputSizes=1
  Selector7 : Selector  InputPortWidth=4  IndexOptions=Index vector (dialog)  Indices=4  OutputSizes=1
  P0x : Outport
  P1x : Outport  Port=2
  P2x : Outport  Port=3
  P3x : Outport  Port=4
  P0y : Outport  Port=5
  P1y : Outport  Port=6
  P2y : Outport  Port=7
  P3y : Outport  Port=8
  wires:
  Bus Selector/2 -> Selector4/1, Selector5/1, Selector6/1, Selector7/1  "<CtrlAryLat>"
  Selector7/1 -> P3y/1  "P3y"
  Selector6/1 -> P2y/1  "P2y"
  Selector5/1 -> P1y/1  "P1y"
  Selector4/1 -> P0y/1  "P0y"
  Selector3/1 -> P3x/1  "P3x"
  Selector2/1 -> P2x/1  "P2x"
  Selector1/1 -> P1x/1  "P1x"
  Selector/1 -> P0x/1  "P0x"
  Bus Selector/1 -> Selector2/1, Selector1/1, Selector/1, Selector3/1  "<CtrlAryLgt>"
  Segment/1 -> Bus Selector/1

== system TosAeb/AEB_Objsel/TA_Processor/AEB_ObjSelect/TargetInfoCheck/Data_precess/TA_EffectCalculate/Obj_FilterFlgCalculate/Long_ObjFilter/TTR_Calculate/CalculateTTRAndTTM_else/LongitudinalTTRComputer/CalculateTTI/HostAndObjectMoving/CheckIfSolutionExists/LimitedSafeDivide/SafeDivide ==
  Numerator : Inport  OutDataTypeStr=DataType
      settings: AttributesFormatString=DT=%<OutDataTypeStr>
  Denominator : Inport  Port=2  OutDataTypeStr=DataType
      settings: AttributesFormatString=DT=%<OutDataTypeStr>
  SafeDivide : MATLAB Function
  Quotient : Outport  OutDataTypeStr=DataType
      settings: AttributesFormatString=DT=%<OutDataTypeStr>
  wires:
  SafeDivide/1(Quotient) -> Quotient/1
  Denominator/1 -> SafeDivide/2(Denominator)  "Denominator"
  Numerator/1 -> SafeDivide/1(Numerator)  "Numerator"

== system TosAeb/AEB_Objsel/TA_Processor/AEB_ObjSelect/TargetInfoCheck/Data_precess/TA_EffectCalculate/Obj_FilterFlgCalculate/Long_ObjFilter/TTR_Calculate/CalculateTTRAndTTM_else/LongitudinalTTRComputer/CalculateTTI/HostAndObjectMoving/CheckIfSolutionExists/LimitedSafeDivide/Saturation Dynamic ==
  up : Inport
  u : Inport  Port=2
  lo : Inport  Port=3
  Data Type Duplicate : DataTypeDuplicate  DisableCoverage=on
  Data Type Propagation : S-Function  FunctionName=sfix_dtprop  Parameters=14,PropDataTypeMode,(getdatatypespecs(PropDataType,PropScaling,DblOver,0, 0, PropDataTypeMode, PropScalingMode)),IfRefDouble,IfRefSingle,IsSigned,NumBitsBase,NumBitsMult,NumBitsAdd,(sort(reshape(round(NumBitsAllowFinal), prod(size(NumBitsAllowFinal)),1))),PropScalingMode,ValuesUsedBestPrec,SlopeBase,SlopeMult,SlopeAdd,BiasBase,BiasMult,BiasAdd,DblOver  SFunctionDeploymentMode=off  EnableBusSupport=off  mask(Data Type Propagation){PropDataTypeMode=Inherit via propagation rule, PropDataType=fixdt(1, 16), IfRefDouble=double, IfRefSingle=single, IsSigned=IsSigned1, NumBitsBase=NumBits1, NumBitsMult=1, NumBitsAdd=0, NumBitsAllowFinal=1:128, PropScalingMode=Inherit via propagation rule, PropScaling=2^-10, ValuesUsedBestPrec=[5 -7], SlopeBase=Slope1, SlopeMult=1, SlopeAdd=0, BiasBase=Bias1, BiasMult=1, BiasAdd=0}
      mask prompts: PropDataTypeMode "Simulink:masks:x1PropagatedDataType_MP"; PropDataType "Simulink:masks:x11PropagatedDataTypeegFixdt116Fixdtsingle_MP"; IfRefDouble "Simulink:masks:x11IfAnyReferenceInputIsDoubleOutputIs_MP"; IfRefSingle "Simulink:masks:x12IfAnyReferenceInputIsSingleOutputIs_MP"; IsSigned "Simulink:masks:x13IsSigned_MP"; NumBitsBase "Simulink:masks:x141NumberofBitsBase_MP"; NumBitsMult "Simulink:masks:x142NumberofBitsMultiplicativeAdjustment_MP"; NumBitsAdd "Simulink:masks:x143NumberofBitsAdditiveAdjustment_MP"; NumBitsAllowFinal "Simulink:masks:x144NumberofBitsAllowableFinalValues_MP"; PropScalingMode "Simulink:masks:x2PropagatedScaling_MP"; PropScaling "Simulink:masks:x21PropagatedScalingSlopeEg29OrSlopeBiasEg1253_MP"; ValuesUsedBestPrec "Simulink:masks:x21ValuesUsedToDetermineBestPrecisionScaling_MP"; SlopeBase "Simulink:masks:x211SlopeBase_MP"; SlopeMult "Simulink:masks:x212SlopeMultiplicativeAdjustment_MP"; SlopeAdd "Simulink:masks:x213SlopeAdditiveAdjustment_MP"; BiasBase "Simulink:masks:x221BiasBase_MP"; BiasMult "Simulink:masks:x222BiasMultiplicativeAdjustment_MP"; BiasAdd "Simulink:masks:x223BiasAdditiveAdjustment_MP"
      mask help: Simulink:masks:DataTypePropagation_MD
      mask init:
      | DblOver = 0;
      mask TypeOptions PropDataTypeMode: Simulink:masks:SpecifyViaDialog | Simulink:masks:InheritViaPropagationRule
      mask Callback PropDataTypeMode: fixptdialog(gcbh)
      mask Callback PropDataType: fixptdialog(gcbh)
      mask TypeOptions IfRefDouble: Simulink:masks:double | Simulink:masks:single
      mask TypeOptions IfRefSingle: Simulink:masks:double | Simulink:masks:single
      mask TypeOptions IsSigned: Simulink:masks:IsSigned1 | Simulink:masks:IsSigned2 | Simulink:masks:IsSigned1OrIsSigned2 | Simulink:masks:TRUE | Simulink:masks:FALSE
      mask TypeOptions NumBitsBase: NumBits1 | NumBits2 | max([NumBits1 NumBits2]) | min([NumBits1 NumBits2]) | NumBits1+NumBits2
      mask TypeOptions PropScalingMode: Simulink:masks:SpecifyViaDialog | Simulink:masks:InheritViaPropagationRule | Simulink:masks:ObtainViaBestPrecision
      mask Callback PropScalingMode: fixptdialog(gcbh)
      mask TypeOptions SlopeBase: Slope1 | Slope2 | max([Slope1 Slope2]) | min([Slope1 Slope2]) | Slope1*Slope2 | Slope1/Slope2 | PosRange1 | PosRange2 | max([PosRange1 PosRange2]) | min([PosRange1 PosRange2]) | PosRange1*PosRange2 | PosRange1/PosRange2
      mask TypeOptions BiasBase: Bias1 | Bias2 | max([Bias1 Bias2]) | min([Bias1 Bias2]) | Bias1*Bias2 | Bias1/Bias2 | Bias1+Bias2 | Bias1-Bias2
      mask Display:
      | port_label('Input',1,'Ref1');
      | port_label('Input',2,'Ref2');
      | port_label('Input',3,'Prop');
  LowerRelop1 : RelationalOperator  DisableCoverage=on  Operator=>  OutDataTypeStr=boolean  RndMeth=Nearest
  Switch : Switch  Criteria=u2 ~= 0  OutDataTypeStr=Inherit: Inherit via back propagation  ZeroCross=off
  Switch2 : Switch  Criteria=u2 ~= 0  OutMin=OutMin  OutMax=OutMax  OutDataTypeStr=Inherit: Inherit via back propagation  ZeroCross=off
  UpperRelop : RelationalOperator  DisableCoverage=on  Operator=<  OutDataTypeStr=boolean  RndMeth=Nearest
  y : Outport  OutMin=OutMin  OutMax=OutMax
  wires:
  up/1 -> Switch2/1, LowerRelop1/2
  u/1 -> Data Type Propagation/2, Data Type Propagation/1, LowerRelop1/1, Switch/3, UpperRelop/1
  lo/1 -> Switch/1, UpperRelop/2
  Switch/1 -> Data Type Duplicate/2, Switch2/3, Data Type Propagation/3
  Switch2/1 -> Data Type Duplicate/1, y/1
  LowerRelop1/1 -> Switch2/2
  UpperRelop/1 -> Switch/2

== system TosAeb/AEB_Objsel/TA_Processor/AEB_ObjSelect/TargetInfoCheck/Data_precess/TA_EffectCalculate/Obj_FilterFlgCalculate/Long_ObjFilter/TTR_Calculate/CalculateTTRAndTTM_else/LongitudinalTTRComputer/CalculateTTI/HostAndObjectMoving/CheckIfSolutionExists1/LimitedSafeDivide/SafeDivide ==
  Numerator : Inport  OutDataTypeStr=DataType
      settings: AttributesFormatString=DT=%<OutDataTypeStr>
  Denominator : Inport  Port=2  OutDataTypeStr=DataType
      settings: AttributesFormatString=DT=%<OutDataTypeStr>
  SafeDivide : MATLAB Function
  Quotient : Outport  OutDataTypeStr=DataType
      settings: AttributesFormatString=DT=%<OutDataTypeStr>
  wires:
  SafeDivide/1(Quotient) -> Quotient/1
  Denominator/1 -> SafeDivide/2(Denominator)  "Denominator"
  Numerator/1 -> SafeDivide/1(Numerator)  "Numerator"

== system TosAeb/AEB_Objsel/TA_Processor/AEB_ObjSelect/TargetInfoCheck/Data_precess/TA_EffectCalculate/Obj_FilterFlgCalculate/Long_ObjFilter/TTR_Calculate/CalculateTTRAndTTM_else/LongitudinalTTRComputer/CalculateTTI/HostAndObjectMoving/CheckIfSolutionExists1/LimitedSafeDivide/Saturation Dynamic ==
  up : Inport
  u : Inport  Port=2
  lo : Inport  Port=3
  Data Type Duplicate : DataTypeDuplicate  DisableCoverage=on
  Data Type Propagation : S-Function  FunctionName=sfix_dtprop  Parameters=14,PropDataTypeMode,(getdatatypespecs(PropDataType,PropScaling,DblOver,0, 0, PropDataTypeMode, PropScalingMode)),IfRefDouble,IfRefSingle,IsSigned,NumBitsBase,NumBitsMult,NumBitsAdd,(sort(reshape(round(NumBitsAllowFinal), prod(size(NumBitsAllowFinal)),1))),PropScalingMode,ValuesUsedBestPrec,SlopeBase,SlopeMult,SlopeAdd,BiasBase,BiasMult,BiasAdd,DblOver  SFunctionDeploymentMode=off  EnableBusSupport=off  mask(Data Type Propagation){PropDataTypeMode=Inherit via propagation rule, PropDataType=fixdt(1, 16), IfRefDouble=double, IfRefSingle=single, IsSigned=IsSigned1, NumBitsBase=NumBits1, NumBitsMult=1, NumBitsAdd=0, NumBitsAllowFinal=1:128, PropScalingMode=Inherit via propagation rule, PropScaling=2^-10, ValuesUsedBestPrec=[5 -7], SlopeBase=Slope1, SlopeMult=1, SlopeAdd=0, BiasBase=Bias1, BiasMult=1, BiasAdd=0}
      mask prompts: PropDataTypeMode "Simulink:masks:x1PropagatedDataType_MP"; PropDataType "Simulink:masks:x11PropagatedDataTypeegFixdt116Fixdtsingle_MP"; IfRefDouble "Simulink:masks:x11IfAnyReferenceInputIsDoubleOutputIs_MP"; IfRefSingle "Simulink:masks:x12IfAnyReferenceInputIsSingleOutputIs_MP"; IsSigned "Simulink:masks:x13IsSigned_MP"; NumBitsBase "Simulink:masks:x141NumberofBitsBase_MP"; NumBitsMult "Simulink:masks:x142NumberofBitsMultiplicativeAdjustment_MP"; NumBitsAdd "Simulink:masks:x143NumberofBitsAdditiveAdjustment_MP"; NumBitsAllowFinal "Simulink:masks:x144NumberofBitsAllowableFinalValues_MP"; PropScalingMode "Simulink:masks:x2PropagatedScaling_MP"; PropScaling "Simulink:masks:x21PropagatedScalingSlopeEg29OrSlopeBiasEg1253_MP"; ValuesUsedBestPrec "Simulink:masks:x21ValuesUsedToDetermineBestPrecisionScaling_MP"; SlopeBase "Simulink:masks:x211SlopeBase_MP"; SlopeMult "Simulink:masks:x212SlopeMultiplicativeAdjustment_MP"; SlopeAdd "Simulink:masks:x213SlopeAdditiveAdjustment_MP"; BiasBase "Simulink:masks:x221BiasBase_MP"; BiasMult "Simulink:masks:x222BiasMultiplicativeAdjustment_MP"; BiasAdd "Simulink:masks:x223BiasAdditiveAdjustment_MP"
      mask help: Simulink:masks:DataTypePropagation_MD
      mask init:
      | DblOver = 0;
      mask TypeOptions PropDataTypeMode: Simulink:masks:SpecifyViaDialog | Simulink:masks:InheritViaPropagationRule
      mask Callback PropDataTypeMode: fixptdialog(gcbh)
      mask Callback PropDataType: fixptdialog(gcbh)
      mask TypeOptions IfRefDouble: Simulink:masks:double | Simulink:masks:single
      mask TypeOptions IfRefSingle: Simulink:masks:double | Simulink:masks:single
      mask TypeOptions IsSigned: Simulink:masks:IsSigned1 | Simulink:masks:IsSigned2 | Simulink:masks:IsSigned1OrIsSigned2 | Simulink:masks:TRUE | Simulink:masks:FALSE
      mask TypeOptions NumBitsBase: NumBits1 | NumBits2 | max([NumBits1 NumBits2]) | min([NumBits1 NumBits2]) | NumBits1+NumBits2
      mask TypeOptions PropScalingMode: Simulink:masks:SpecifyViaDialog | Simulink:masks:InheritViaPropagationRule | Simulink:masks:ObtainViaBestPrecision
      mask Callback PropScalingMode: fixptdialog(gcbh)
      mask TypeOptions SlopeBase: Slope1 | Slope2 | max([Slope1 Slope2]) | min([Slope1 Slope2]) | Slope1*Slope2 | Slope1/Slope2 | PosRange1 | PosRange2 | max([PosRange1 PosRange2]) | min([PosRange1 PosRange2]) | PosRange1*PosRange2 | PosRange1/PosRange2
      mask TypeOptions BiasBase: Bias1 | Bias2 | max([Bias1 Bias2]) | min([Bias1 Bias2]) | Bias1*Bias2 | Bias1/Bias2 | Bias1+Bias2 | Bias1-Bias2
      mask Display:
      | port_label('Input',1,'Ref1');
      | port_label('Input',2,'Ref2');
      | port_label('Input',3,'Prop');
  LowerRelop1 : RelationalOperator  DisableCoverage=on  Operator=>  OutDataTypeStr=boolean  RndMeth=Nearest
  Switch : Switch  Criteria=u2 ~= 0  OutDataTypeStr=Inherit: Inherit via back propagation  ZeroCross=off
  Switch2 : Switch  Criteria=u2 ~= 0  OutMin=OutMin  OutMax=OutMax  OutDataTypeStr=Inherit: Inherit via back propagation  ZeroCross=off
  UpperRelop : RelationalOperator  DisableCoverage=on  Operator=<  OutDataTypeStr=boolean  RndMeth=Nearest
  y : Outport  OutMin=OutMin  OutMax=OutMax
  wires:
  up/1 -> Switch2/1, LowerRelop1/2
  u/1 -> Data Type Propagation/2, Data Type Propagation/1, LowerRelop1/1, Switch/3, UpperRelop/1
  lo/1 -> Switch/1, UpperRelop/2
  Switch/1 -> Data Type Duplicate/2, Switch2/3, Data Type Propagation/3
  Switch2/1 -> Data Type Duplicate/1, y/1
  LowerRelop1/1 -> Switch2/2
  UpperRelop/1 -> Switch/2

== system TosAeb/AEB_Objsel/TA_Processor/AEB_ObjSelect/TargetInfoCheck/Data_precess/TA_EffectCalculate/Obj_FilterFlgCalculate/Long_ObjFilter/TTR_Calculate/CalculateTTRAndTTM_else/LongitudinalTTRComputer/CalculateTTI/HostAndObjectMoving/TimeToIntersectionComputer/SolutionChooser/Compare To Zero1 ==
  u : Inport
  Compare : RelationalOperator  Operator=<  OutDataTypeStr=boolean  RndMeth=Nearest  InputSameDT=on
  Constant : Constant  DisableCoverage=on  Value=0  OutDataTypeStr=Inherit: Inherit via back propagation  PreserveConstantTs=on
  y : Outport
  wires:
  Constant/1 -> Compare/2
  u/1 -> Compare/1
  Compare/1 -> y/1

== system TosAeb/AEB_Objsel/TA_Processor/AEB_ObjSelect/TargetInfoCheck/Data_precess/TA_EffectCalculate/Obj_FilterFlgCalculate/Long_ObjFilter/TTR_Calculate/CalculateTTRAndTTM_else/LongitudinalTTRComputer/CalculateTTI/HostAndObjectMoving/TimeToIntersectionComputer1/SolutionChooser/Compare To Zero1 ==
  u : Inport
  Compare : RelationalOperator  Operator=<  OutDataTypeStr=boolean  RndMeth=Nearest  InputSameDT=on
  Constant : Constant  DisableCoverage=on  Value=0  OutDataTypeStr=Inherit: Inherit via back propagation  PreserveConstantTs=on
  y : Outport
  wires:
  Compare/1 -> y/1
  u/1 -> Compare/1
  Constant/1 -> Compare/2

== system TosAeb/AEB_Objsel/TA_Processor/AEB_ObjSelect/TargetInfoCheck/Data_precess/TA_EffectCalculate/Obj_FilterFlgCalculate/Long_ObjFilter/TTR_Calculate/CalculateTTRAndTTM_else/LongitudinalTTRComputer/CalculateTTI/StationaryHost/CheckIfSolutionExists/LimitedSafeDivide/SafeDivide ==
  Numerator : Inport  OutDataTypeStr=DataType
      settings: AttributesFormatString=DT=%<OutDataTypeStr>
  Denominator : Inport  Port=2  OutDataTypeStr=DataType
      settings: AttributesFormatString=DT=%<OutDataTypeStr>
  SafeDivide : MATLAB Function
  Quotient : Outport  OutDataTypeStr=DataType
      settings: AttributesFormatString=DT=%<OutDataTypeStr>
  wires:
  SafeDivide/1(Quotient) -> Quotient/1
  Denominator/1 -> SafeDivide/2(Denominator)  "Denominator"
  Numerator/1 -> SafeDivide/1(Numerator)  "Numerator"
